simulink model "os_polyphase_synth_4096c"  (R2021a) (continued)
== system os_polyphase_synth_4096c/pfb_fir/pol1_in1_tap77 == (continued)
      | -PCIN>>17+P+(D-A)*(D-A)
      | -PCIN>>17+P+(D-A)*B
      | -PCIN>>17+P+(D-A)*BCIN
      | -PCIN>>17+P+(D-ACIN)
      | -PCIN>>17+P+(D-ACIN)*(D-ACIN)
      | -PCIN>>17+P+(D-ACIN)*B
      | -PCIN>>17+P+(D-ACIN)*BCIN
      | -PCIN>>17+P+A
      | -PCIN>>17+P+A*A
      | -PCIN>>17+P+A*B
      | -PCIN>>17+P+A*BCIN
      | -PCIN>>17+P+ACIN
      | -PCIN>>17+P+ACIN*ACIN
      | -PCIN>>17+P+ACIN*B
      | -PCIN>>17+P+ACIN*BCIN
      | -PCIN>>17+P+B*(A+D)
      | -PCIN>>17+P+B*(ACIN+D)
      | -PCIN>>17+P+B*(D+A)
      | -PCIN>>17+P+B*(D+ACIN)
      | -PCIN>>17+P+B*(D-A)
      | -PCIN>>17+P+B*(D-ACIN)
      | -PCIN>>17+P+B*A
      | -PCIN>>17+P+B*ACIN
      | -PCIN>>17+P+B*D
      | -PCIN>>17+P+BCIN*(A+D)
      | -PCIN>>17+P+BCIN*(ACIN+D)
      | -PCIN>>17+P+BCIN*(D+A)
      | -PCIN>>17+P+BCIN*(D+ACIN)
      | -PCIN>>17+P+BCIN*(D-A)
      | -PCIN>>17+P+BCIN*(D-ACIN)
      | -PCIN>>17+P+BCIN*A
      | -PCIN>>17+P+BCIN*ACIN
      | -PCIN>>17+P+BCIN*D
      | -PCIN>>17+P+C
      | -PCIN>>17+P+C+C
      | -PCIN>>17+P+C+CONCAT
      | -PCIN>>17+P+C+P
      | -PCIN>>17+P+CONCAT
      | -PCIN>>17+P+CONCAT+C
      | -PCIN>>17+P+D
      | -PCIN>>17+P+D*B
      | -PCIN>>17+P+D*BCIN
      | -PCIN>>17+P+D*D
      | -PCIN>>17+P+P
      | -PCIN>>17+P+P+C
      | -PCIN>>17-((A+D))
      | -PCIN>>17-((A+D)*(A+D))
      | -PCIN>>17-((A+D)*B)
      | -PCIN>>17-((A+D)*BCIN)
      | -PCIN>>17-((ACIN+D))
      | -PCIN>>17-((ACIN+D)*(ACIN+D))
      | -PCIN>>17-((ACIN+D)*B)
      | -PCIN>>17-((ACIN+D)*BCIN)
      | -PCIN>>17-((D+A))
      | -PCIN>>17-((D+A)*(D+A))
      | -PCIN>>17-((D+A)*B)
      | -PCIN>>17-((D+A)*BCIN)
      | -PCIN>>17-((D+ACIN))
      | -PCIN>>17-((D+ACIN)*(D+ACIN))
      | -PCIN>>17-((D+ACIN)*B)
      | -PCIN>>17-((D+ACIN)*BCIN)
      | -PCIN>>17-((D-A))
      | -PCIN>>17-((D-A)*(D-A))
      | -PCIN>>17-((D-A)*B)
      | -PCIN>>17-((D-A)*BCIN)
      | -PCIN>>17-((D-ACIN))
      | -PCIN>>17-((D-ACIN)*(D-ACIN))
      | -PCIN>>17-((D-ACIN)*B)
      | -PCIN>>17-((D-ACIN)*BCIN)
      | -PCIN>>17-(A)
      | -PCIN>>17-(A*A)
      | -PCIN>>17-(A*B)
      | -PCIN>>17-(A*BCIN)
      | -PCIN>>17-(ACIN)
      | -PCIN>>17-(ACIN*ACIN)
      | -PCIN>>17-(ACIN*B)
      | -PCIN>>17-(ACIN*BCIN)
      | -PCIN>>17-(B*(A+D))
      | -PCIN>>17-(B*(ACIN+D))
      | -PCIN>>17-(B*(D+A))
      | -PCIN>>17-(B*(D+ACIN))
      | -PCIN>>17-(B*(D-A))
      | -PCIN>>17-(B*(D-ACIN))
      | -PCIN>>17-(B*A)
      | -PCIN>>17-(B*ACIN)
      | -PCIN>>17-(B*D)
      | -PCIN>>17-(BCIN*(A+D))
      | -PCIN>>17-(BCIN*(ACIN+D))
      | -PCIN>>17-(BCIN*(D+A))
      | -PCIN>>17-(BCIN*(D+ACIN))
      | -PCIN>>17-(BCIN*(D-A))
      | -PCIN>>17-(BCIN*(D-ACIN))
      | -PCIN>>17-(BCIN*A)
      | -PCIN>>17-(BCIN*ACIN)
      | -PCIN>>17-(BCIN*D)
      | -PCIN>>17-(D)
      | -PCIN>>17-(D*B)
      | -PCIN>>17-(D*BCIN)
      | -PCIN>>17-(D*D)
      | 0
      | A
      | A*A
      | A*A+C
      | A*A+C+C
      | A*A+C+C+CARRYIN
      | A*A+C+CARRYIN
      | A*A+C+P
      | A*A+C+P+CARRYIN
      | A*A+C+P>>17
      | A*A+C+P>>17+CARRYIN
      | A*A+C+PCIN
      | A*A+C+PCIN+CARRYIN
      | A*A+C+PCIN>>17
      | A*A+C+PCIN>>17+CARRYIN
      | A*A+C-C
      | A*A+C-P
      | A*A+C-P>>17
      | A*A+C-PCIN
      | A*A+C-PCIN>>17
      | A*A+CARRYIN
      | A*A+P
      | A*A+P+C
      | A*A+P+C+CARRYIN
      | A*A+P+CARRYIN
      | A*A+P+P
      | A*A+P+P+CARRYIN
      | A*A+P+P>>17
      | A*A+P+P>>17+CARRYIN
      | A*A+P+PCIN
      | A*A+P+PCIN+CARRYIN
      | A*A+P+PCIN>>17
      | A*A+P+PCIN>>17+CARRYIN
      | A*A+P-C
      | A*A+P-P
      | A*A+P-P>>17
      | A*A+P-PCIN
      | A*A+P-PCIN>>17
      | A*A+P>>17
      | A*A+P>>17+C
      | A*A+P>>17+C+CARRYIN
      | A*A+P>>17+CARRYIN
      | A*A+P>>17+P
      | A*A+P>>17+P+CARRYIN
      | A*A+PCIN
      | A*A+PCIN+C
      | A*A+PCIN+C+CARRYIN
      | A*A+PCIN+CARRYIN
      | A*A+PCIN+P
      | A*A+PCIN+P+CARRYIN
      | A*A+PCIN>>17
      | A*A+PCIN>>17+C
      | A*A+PCIN>>17+C+CARRYIN
      | A*A+PCIN>>17+CARRYIN
      | A*A+PCIN>>17+P
      | A*A+PCIN>>17+P+CARRYIN
      | A*A-C
      | A*A-P
      | A*A-P>>17
      | A*A-PCIN
      | A*A-PCIN>>17
      | A*B
      | A*B+C
      | A*B+C+C
      | A*B+C+C+CARRYIN
      | A*B+C+CARRYIN
      | A*B+C+P
      | A*B+C+P+CARRYIN
      | A*B+C+P>>17
      | A*B+C+P>>17+CARRYIN
      | A*B+C+PCIN
      | A*B+C+PCIN+CARRYIN
      | A*B+C+PCIN>>17
      | A*B+C+PCIN>>17+CARRYIN
      | A*B+C-C
      | A*B+C-P
      | A*B+C-P>>17
      | A*B+C-PCIN
      | A*B+C-PCIN>>17
      | A*B+CARRYIN
      | A*B+P
      | A*B+P+C
      | A*B+P+C+CARRYIN
      | A*B+P+CARRYIN
      | A*B+P+P
      | A*B+P+P+CARRYIN
      | A*B+P+P>>17
      | A*B+P+P>>17+CARRYIN
      | A*B+P+PCIN
      | A*B+P+PCIN+CARRYIN
      | A*B+P+PCIN>>17
      | A*B+P+PCIN>>17+CARRYIN
      | A*B+P-C
      | A*B+P-P
      | A*B+P-P>>17
      | A*B+P-PCIN
      | A*B+P-PCIN>>17
      | A*B+P>>17
      | A*B+P>>17+C
      | A*B+P>>17+C+CARRYIN
      | A*B+P>>17+CARRYIN
      | A*B+P>>17+P
      | A*B+P>>17+P+CARRYIN
      | A*B+PCIN
      | A*B+PCIN+C
      | A*B+PCIN+C+CARRYIN
      | A*B+PCIN+CARRYIN
      | A*B+PCIN+P
      | A*B+PCIN+P+CARRYIN
      | A*B+PCIN>>17
      | A*B+PCIN>>17+C
      | A*B+PCIN>>17+C+CARRYIN
      | A*B+PCIN>>17+CARRYIN
      | A*B+PCIN>>17+P
      | A*B+PCIN>>17+P+CARRYIN
      | A*B-C
      | A*B-P
      | A*B-P>>17
      | A*B-PCIN
      | A*B-PCIN>>17
      | A*BCIN
      | A*BCIN+C
      | A*BCIN+C+C
      | A*BCIN+C+C+CARRYIN
      | A*BCIN+C+CARRYIN
      | A*BCIN+C+P
      | A*BCIN+C+P+CARRYIN
      | A*BCIN+C+P>>17
      | A*BCIN+C+P>>17+CARRYIN
      | A*BCIN+C+PCIN
      | A*BCIN+C+PCIN+CARRYIN
      | A*BCIN+C+PCIN>>17
      | A*BCIN+C+PCIN>>17+CARRYIN
      | A*BCIN+C-C
      | A*BCIN+C-P
      | A*BCIN+C-P>>17
      | A*BCIN+C-PCIN
      | A*BCIN+C-PCIN>>17
      | A*BCIN+CARRYIN
      | A*BCIN+P
      | A*BCIN+P+C
      | A*BCIN+P+C+CARRYIN
      | A*BCIN+P+CARRYIN
      | A*BCIN+P+P
      | A*BCIN+P+P+CARRYIN
      | A*BCIN+P+P>>17
      | A*BCIN+P+P>>17+CARRYIN
      | A*BCIN+P+PCIN
      | A*BCIN+P+PCIN+CARRYIN
      | A*BCIN+P+PCIN>>17
      | A*BCIN+P+PCIN>>17+CARRYIN
      | A*BCIN+P-C
      | A*BCIN+P-P
      | A*BCIN+P-P>>17
      | A*BCIN+P-PCIN
      | A*BCIN+P-PCIN>>17
      | A*BCIN+P>>17
      | A*BCIN+P>>17+C
      | A*BCIN+P>>17+C+CARRYIN
      | A*BCIN+P>>17+CARRYIN
      | A*BCIN+P>>17+P
      | A*BCIN+P>>17+P+CARRYIN
      | A*BCIN+PCIN
      | A*BCIN+PCIN+C
      | A*BCIN+PCIN+C+CARRYIN
      | A*BCIN+PCIN+CARRYIN
      | A*BCIN+PCIN+P
      | A*BCIN+PCIN+P+CARRYIN
      | A*BCIN+PCIN>>17
      | A*BCIN+PCIN>>17+C
      | A*BCIN+PCIN>>17+C+CARRYIN
      | A*BCIN+PCIN>>17+CARRYIN
      | A*BCIN+PCIN>>17+P
      | A*BCIN+PCIN>>17+P+CARRYIN
      | A*BCIN-C
      | A*BCIN-P
      | A*BCIN-P>>17
      | A*BCIN-PCIN
      | A*BCIN-PCIN>>17
      | A+C
      | A+C+C
      | A+C+C+CARRYIN
      | A+C+CARRYIN
      | A+C+P
      | A+C+P+CARRYIN
      | A+C+P>>17
      | A+C+P>>17+CARRYIN
      | A+C+PCIN
      | A+C+PCIN+CARRYIN
      | A+C+PCIN>>17
      | A+C+PCIN>>17+CARRYIN
      | A+C-C
      | A+C-P
      | A+C-P>>17
      | A+C-PCIN
      | A+C-PCIN>>17
      | A+CARRYIN
      | A+P
      | A+P+C
      | A+P+C+CARRYIN
      | A+P+CARRYIN
      | A+P+P
      | A+P+P+CARRYIN
      | A+P+P>>17
      | A+P+P>>17+CARRYIN
      | A+P+PCIN
      | A+P+PCIN+CARRYIN
      | A+P+PCIN>>17
      | A+P+PCIN>>17+CARRYIN
      | A+P-C
      | A+P-P
      | A+P-P>>17
      | A+P-PCIN
      | A+P-PCIN>>17
      | A+P>>17
      | A+P>>17+C
      | A+P>>17+C+CARRYIN
      | A+P>>17+CARRYIN
      | A+P>>17+P
      | A+P>>17+P+CARRYIN
      | A+PCIN
      | A+PCIN+C
      | A+PCIN+C+CARRYIN
      | A+PCIN+CARRYIN
      | A+PCIN+P
      | A+PCIN+P+CARRYIN
      | A+PCIN>>17
      | A+PCIN>>17+C
      | A+PCIN>>17+C+CARRYIN
      | A+PCIN>>17+CARRYIN
      | A+PCIN>>17+P
      | A+PCIN>>17+P+CARRYIN
      | A-C
      | A-P
      | A-P>>17
      | A-PCIN
      | A-PCIN>>17
      | ACIN
      | ACIN*ACIN
      | ACIN*ACIN+C
      | ACIN*ACIN+C+C
      | ACIN*ACIN+C+C+CARRYIN
      | ACIN*ACIN+C+CARRYIN
      | ACIN*ACIN+C+P
      | ACIN*ACIN+C+P+CARRYIN
      | ACIN*ACIN+C+P>>17
      | ACIN*ACIN+C+P>>17+CARRYIN
      | ACIN*ACIN+C+PCIN
      | ACIN*ACIN+C+PCIN+CARRYIN
      | ACIN*ACIN+C+PCIN>>17
      | ACIN*ACIN+C+PCIN>>17+CARRYIN
      | ACIN*ACIN+C-C
      | ACIN*ACIN+C-P
      | ACIN*ACIN+C-P>>17
      | ACIN*ACIN+C-PCIN
      | ACIN*ACIN+C-PCIN>>17
      | ACIN*ACIN+CARRYIN
      | ACIN*ACIN+P
      | ACIN*ACIN+P+C
      | ACIN*ACIN+P+C+CARRYIN
      | ACIN*ACIN+P+CARRYIN
      | ACIN*ACIN+P+P
      | ACIN*ACIN+P+P+CARRYIN
      | ACIN*ACIN+P+P>>17
      | ACIN*ACIN+P+P>>17+CARRYIN
      | ACIN*ACIN+P+PCIN
      | ACIN*ACIN+P+PCIN+CARRYIN
      | ACIN*ACIN+P+PCIN>>17
      | ACIN*ACIN+P+PCIN>>17+CARRYIN
      | ACIN*ACIN+P-C
      | ACIN*ACIN+P-P
      | ACIN*ACIN+P-P>>17
      | ACIN*ACIN+P-PCIN
      | ACIN*ACIN+P-PCIN>>17
      | ACIN*ACIN+P>>17
      | ACIN*ACIN+P>>17+C
      | ACIN*ACIN+P>>17+C+CARRYIN
      | ACIN*ACIN+P>>17+CARRYIN
      | ACIN*ACIN+P>>17+P
      | ACIN*ACIN+P>>17+P+CARRYIN
      | ACIN*ACIN+PCIN
      | ACIN*ACIN+PCIN+C
      | ACIN*ACIN+PCIN+C+CARRYIN
      | ACIN*ACIN+PCIN+CARRYIN
      | ACIN*ACIN+PCIN+P
      | ACIN*ACIN+PCIN+P+CARRYIN
      | ACIN*ACIN+PCIN>>17
      | ACIN*ACIN+PCIN>>17+C
      | ACIN*ACIN+PCIN>>17+C+CARRYIN
      | ACIN*ACIN+PCIN>>17+CARRYIN
      | ACIN*ACIN+PCIN>>17+P
      | ACIN*ACIN+PCIN>>17+P+CARRYIN
      | ACIN*ACIN-C
      | ACIN*ACIN-P
      | ACIN*ACIN-P>>17
      | ACIN*ACIN-PCIN
      | ACIN*ACIN-PCIN>>17
      | ACIN*B
      | ACIN*B+C
      | ACIN*B+C+C
      | ACIN*B+C+C+CARRYIN
      | ACIN*B+C+CARRYIN
      | ACIN*B+C+P
      | ACIN*B+C+P+CARRYIN
      | ACIN*B+C+P>>17
      | ACIN*B+C+P>>17+CARRYIN
      | ACIN*B+C+PCIN
      | ACIN*B+C+PCIN+CARRYIN
      | ACIN*B+C+PCIN>>17
      | ACIN*B+C+PCIN>>17+CARRYIN
      | ACIN*B+C-C
      | ACIN*B+C-P
      | ACIN*B+C-P>>17
      | ACIN*B+C-PCIN
      | ACIN*B+C-PCIN>>17
      | ACIN*B+CARRYIN
      | ACIN*B+P
      | ACIN*B+P+C
      | ACIN*B+P+C+CARRYIN
      | ACIN*B+P+CARRYIN
      | ACIN*B+P+P
      | ACIN*B+P+P+CARRYIN
      | ACIN*B+P+P>>17
      | ACIN*B+P+P>>17+CARRYIN
      | ACIN*B+P+PCIN
      | ACIN*B+P+PCIN+CARRYIN
      | ACIN*B+P+PCIN>>17
      | ACIN*B+P+PCIN>>17+CARRYIN
      | ACIN*B+P-C
      | ACIN*B+P-P
      | ACIN*B+P-P>>17
      | ACIN*B+P-PCIN
      | ACIN*B+P-PCIN>>17
      | ACIN*B+P>>17
      | ACIN*B+P>>17+C
      | ACIN*B+P>>17+C+CARRYIN
      | ACIN*B+P>>17+CARRYIN
      | ACIN*B+P>>17+P
      | ACIN*B+P>>17+P+CARRYIN
      | ACIN*B+PCIN
      | ACIN*B+PCIN+C
      | ACIN*B+PCIN+C+CARRYIN
      | ACIN*B+PCIN+CARRYIN
      | ACIN*B+PCIN+P
      | ACIN*B+PCIN+P+CARRYIN
      | ACIN*B+PCIN>>17
      | ACIN*B+PCIN>>17+C
      | ACIN*B+PCIN>>17+C+CARRYIN
      | ACIN*B+PCIN>>17+CARRYIN
      | ACIN*B+PCIN>>17+P
      | ACIN*B+PCIN>>17+P+CARRYIN
      | ACIN*B-C
      | ACIN*B-P
      | ACIN*B-P>>17
      | ACIN*B-PCIN
      | ACIN*B-PCIN>>17
      | ACIN*BCIN
      | ACIN*BCIN+C
      | ACIN*BCIN+C+C
      | ACIN*BCIN+C+C+CARRYIN
      | ACIN*BCIN+C+CARRYIN
      | ACIN*BCIN+C+P
      | ACIN*BCIN+C+P+CARRYIN
      | ACIN*BCIN+C+P>>17
      | ACIN*BCIN+C+P>>17+CARRYIN
      | ACIN*BCIN+C+PCIN
      | ACIN*BCIN+C+PCIN+CARRYIN
      | ACIN*BCIN+C+PCIN>>17
      | ACIN*BCIN+C+PCIN>>17+CARRYIN
      | ACIN*BCIN+C-C
      | ACIN*BCIN+C-P
      | ACIN*BCIN+C-P>>17
      | ACIN*BCIN+C-PCIN
      | ACIN*BCIN+C-PCIN>>17
      | ACIN*BCIN+CARRYIN
      | ACIN*BCIN+P
      | ACIN*BCIN+P+C
      | ACIN*BCIN+P+C+CARRYIN
      | ACIN*BCIN+P+CARRYIN
      | ACIN*BCIN+P+P
      | ACIN*BCIN+P+P+CARRYIN
      | ACIN*BCIN+P+P>>17
      | ACIN*BCIN+P+P>>17+CARRYIN
      | ACIN*BCIN+P+PCIN
      | ACIN*BCIN+P+PCIN+CARRYIN
      | ACIN*BCIN+P+PCIN>>17
      | ACIN*BCIN+P+PCIN>>17+CARRYIN
      | ACIN*BCIN+P-C
      | ACIN*BCIN+P-P
      | ACIN*BCIN+P-P>>17
      | ACIN*BCIN+P-PCIN
      | ACIN*BCIN+P-PCIN>>17
      | ACIN*BCIN+P>>17
      | ACIN*BCIN+P>>17+C
      | ACIN*BCIN+P>>17+C+CARRYIN
      | ACIN*BCIN+P>>17+CARRYIN
      | ACIN*BCIN+P>>17+P
      | ACIN*BCIN+P>>17+P+CARRYIN
      | ACIN*BCIN+PCIN
      | ACIN*BCIN+PCIN+C
      | ACIN*BCIN+PCIN+C+CARRYIN
      | ACIN*BCIN+PCIN+CARRYIN
      | ACIN*BCIN+PCIN+P
      | ACIN*BCIN+PCIN+P+CARRYIN
      | ACIN*BCIN+PCIN>>17
      | ACIN*BCIN+PCIN>>17+C
      | ACIN*BCIN+PCIN>>17+C+CARRYIN
      | ACIN*BCIN+PCIN>>17+CARRYIN
      | ACIN*BCIN+PCIN>>17+P
      | ACIN*BCIN+PCIN>>17+P+CARRYIN
      | ACIN*BCIN-C
      | ACIN*BCIN-P
      | ACIN*BCIN-P>>17
      | ACIN*BCIN-PCIN
      | ACIN*BCIN-PCIN>>17
      | ACIN+C
      | ACIN+C+C
      | ACIN+C+C+CARRYIN
      | ACIN+C+CARRYIN
      | ACIN+C+P
      | ACIN+C+P+CARRYIN
      | ACIN+C+P>>17
      | ACIN+C+P>>17+CARRYIN
      | ACIN+C+PCIN
      | ACIN+C+PCIN+CARRYIN
      | ACIN+C+PCIN>>17
      | ACIN+C+PCIN>>17+CARRYIN
      | ACIN+C-C
      | ACIN+C-P
      | ACIN+C-P>>17
      | ACIN+C-PCIN
      | ACIN+C-PCIN>>17
      | ACIN+CARRYIN
      | ACIN+P
      | ACIN+P+C
      | ACIN+P+C+CARRYIN
      | ACIN+P+CARRYIN
      | ACIN+P+P
      | ACIN+P+P+CARRYIN
      | ACIN+P+P>>17
      | ACIN+P+P>>17+CARRYIN
      | ACIN+P+PCIN
      | ACIN+P+PCIN+CARRYIN
      | ACIN+P+PCIN>>17
      | ACIN+P+PCIN>>17+CARRYIN
      | ACIN+P-C
      | ACIN+P-P
      | ACIN+P-P>>17
      | ACIN+P-PCIN
      | ACIN+P-PCIN>>17
      | ACIN+P>>17
      | ACIN+P>>17+C
      | ACIN+P>>17+C+CARRYIN
      | ACIN+P>>17+CARRYIN
      | ACIN+P>>17+P
      | ACIN+P>>17+P+CARRYIN
      | ACIN+PCIN
      | ACIN+PCIN+C
      | ACIN+PCIN+C+CARRYIN
      | ACIN+PCIN+CARRYIN
      | ACIN+PCIN+P
      | ACIN+PCIN+P+CARRYIN
      | ACIN+PCIN>>17
      | ACIN+PCIN>>17+C
      | ACIN+PCIN>>17+C+CARRYIN
      | ACIN+PCIN>>17+CARRYIN
      | ACIN+PCIN>>17+P
      | ACIN+PCIN>>17+P+CARRYIN
      | ACIN-C
      | ACIN-P
      | ACIN-P>>17
      | ACIN-PCIN
      | ACIN-PCIN>>17
      | B*(A+D)
      | B*(A+D)+C
      | B*(A+D)+C+C
      | B*(A+D)+C+C+CARRYIN
      | B*(A+D)+C+CARRYIN
      | B*(A+D)+C+P
      | B*(A+D)+C+P+CARRYIN
      | B*(A+D)+C+P>>17
      | B*(A+D)+C+P>>17+CARRYIN
      | B*(A+D)+C+PCIN
      | B*(A+D)+C+PCIN+CARRYIN
      | B*(A+D)+C+PCIN>>17
      | B*(A+D)+C+PCIN>>17+CARRYIN
      | B*(A+D)+C-C
      | B*(A+D)+C-P
      | B*(A+D)+C-P>>17
      | B*(A+D)+C-PCIN
      | B*(A+D)+C-PCIN>>17
      | B*(A+D)+CARRYIN
      | B*(A+D)+P
      | B*(A+D)+P+C
      | B*(A+D)+P+C+CARRYIN
      | B*(A+D)+P+CARRYIN
      | B*(A+D)+P+P
      | B*(A+D)+P+P+CARRYIN
      | B*(A+D)+P+P>>17
      | B*(A+D)+P+P>>17+CARRYIN
      | B*(A+D)+P+PCIN
      | B*(A+D)+P+PCIN+CARRYIN
      | B*(A+D)+P+PCIN>>17
      | B*(A+D)+P+PCIN>>17+CARRYIN
      | B*(A+D)+P-C
      | B*(A+D)+P-P
      | B*(A+D)+P-P>>17
      | B*(A+D)+P-PCIN
      | B*(A+D)+P-PCIN>>17
      | B*(A+D)+P>>17
      | B*(A+D)+P>>17+C
      | B*(A+D)+P>>17+C+CARRYIN
      | B*(A+D)+P>>17+CARRYIN
      | B*(A+D)+P>>17+P
      | B*(A+D)+P>>17+P+CARRYIN
      | B*(A+D)+PCIN
      | B*(A+D)+PCIN+C
      | B*(A+D)+PCIN+C+CARRYIN
      | B*(A+D)+PCIN+CARRYIN
      | B*(A+D)+PCIN+P
      | B*(A+D)+PCIN+P+CARRYIN
      | B*(A+D)+PCIN>>17
      | B*(A+D)+PCIN>>17+C
      | B*(A+D)+PCIN>>17+C+CARRYIN
      | B*(A+D)+PCIN>>17+CARRYIN
      | B*(A+D)+PCIN>>17+P
      | B*(A+D)+PCIN>>17+P+CARRYIN
      | B*(A+D)-C
      | B*(A+D)-P
      | B*(A+D)-P>>17
      | B*(A+D)-PCIN
      | B*(A+D)-PCIN>>17
      | B*(ACIN+D)
      | B*(ACIN+D)+C
      | B*(ACIN+D)+C+C
      | B*(ACIN+D)+C+C+CARRYIN
      | B*(ACIN+D)+C+CARRYIN
      | B*(ACIN+D)+C+P
      | B*(ACIN+D)+C+P+CARRYIN
      | B*(ACIN+D)+C+P>>17
      | B*(ACIN+D)+C+P>>17+CARRYIN
      | B*(ACIN+D)+C+PCIN
      | B*(ACIN+D)+C+PCIN+CARRYIN
      | B*(ACIN+D)+C+PCIN>>17
      | B*(ACIN+D)+C+PCIN>>17+CARRYIN
      | B*(ACIN+D)+C-C
      | B*(ACIN+D)+C-P
      | B*(ACIN+D)+C-P>>17
      | B*(ACIN+D)+C-PCIN
      | B*(ACIN+D)+C-PCIN>>17
      | B*(ACIN+D)+CARRYIN
      | B*(ACIN+D)+P
      | B*(ACIN+D)+P+C
      | B*(ACIN+D)+P+C+CARRYIN
      | B*(ACIN+D)+P+CARRYIN
      | B*(ACIN+D)+P+P
      | B*(ACIN+D)+P+P+CARRYIN
      | B*(ACIN+D)+P+P>>17
      | B*(ACIN+D)+P+P>>17+CARRYIN
      | B*(ACIN+D)+P+PCIN
      | B*(ACIN+D)+P+PCIN+CARRYIN
      | B*(ACIN+D)+P+PCIN>>17
      | B*(ACIN+D)+P+PCIN>>17+CARRYIN
      | B*(ACIN+D)+P-C
      | B*(ACIN+D)+P-P
      | B*(ACIN+D)+P-P>>17
      | B*(ACIN+D)+P-PCIN
      | B*(ACIN+D)+P-PCIN>>17
      | B*(ACIN+D)+P>>17
      | B*(ACIN+D)+P>>17+C
      | B*(ACIN+D)+P>>17+C+CARRYIN
      | B*(ACIN+D)+P>>17+CARRYIN
      | B*(ACIN+D)+P>>17+P
      | B*(ACIN+D)+P>>17+P+CARRYIN
      | B*(ACIN+D)+PCIN
      | B*(ACIN+D)+PCIN+C
      | B*(ACIN+D)+PCIN+C+CARRYIN
      | B*(ACIN+D)+PCIN+CARRYIN
      | B*(ACIN+D)+PCIN+P
      | B*(ACIN+D)+PCIN+P+CARRYIN
      | B*(ACIN+D)+PCIN>>17
      | B*(ACIN+D)+PCIN>>17+C
      | B*(ACIN+D)+PCIN>>17+C+CARRYIN
      | B*(ACIN+D)+PCIN>>17+CARRYIN
      | B*(ACIN+D)+PCIN>>17+P
      | B*(ACIN+D)+PCIN>>17+P+CARRYIN
      | B*(ACIN+D)-C
      | B*(ACIN+D)-P
      | B*(ACIN+D)-P>>17
      | B*(ACIN+D)-PCIN
      | B*(ACIN+D)-PCIN>>17
      | B*(D+A)
      | B*(D+A)+C
      | B*(D+A)+C+C
      | B*(D+A)+C+C+CARRYIN
      | B*(D+A)+C+CARRYIN
      | B*(D+A)+C+P
      | B*(D+A)+C+P+CARRYIN
      | B*(D+A)+C+P>>17
      | B*(D+A)+C+P>>17+CARRYIN
      | B*(D+A)+C+PCIN
      | B*(D+A)+C+PCIN+CARRYIN
      | B*(D+A)+C+PCIN>>17
      | B*(D+A)+C+PCIN>>17+CARRYIN
      | B*(D+A)+C-C
      | B*(D+A)+C-P
      | B*(D+A)+C-P>>17
      | B*(D+A)+C-PCIN
      | B*(D+A)+C-PCIN>>17
      | B*(D+A)+CARRYIN
      | B*(D+A)+P
      | B*(D+A)+P+C
      | B*(D+A)+P+C+CARRYIN
      | B*(D+A)+P+CARRYIN
      | B*(D+A)+P+P
      | B*(D+A)+P+P+CARRYIN
      | B*(D+A)+P+P>>17
      | B*(D+A)+P+P>>17+CARRYIN
      | B*(D+A)+P+PCIN
      | B*(D+A)+P+PCIN+CARRYIN
      | B*(D+A)+P+PCIN>>17
      | B*(D+A)+P+PCIN>>17+CARRYIN
      | B*(D+A)+P-C
      | B*(D+A)+P-P
      | B*(D+A)+P-P>>17
      | B*(D+A)+P-PCIN
      | B*(D+A)+P-PCIN>>17
      | B*(D+A)+P>>17
      | B*(D+A)+P>>17+C
      | B*(D+A)+P>>17+C+CARRYIN
      | B*(D+A)+P>>17+CARRYIN
      | B*(D+A)+P>>17+P
      | B*(D+A)+P>>17+P+CARRYIN
      | B*(D+A)+PCIN
      | B*(D+A)+PCIN+C
      | B*(D+A)+PCIN+C+CARRYIN
      | B*(D+A)+PCIN+CARRYIN
      | B*(D+A)+PCIN+P
      | B*(D+A)+PCIN+P+CARRYIN
      | B*(D+A)+PCIN>>17
      | B*(D+A)+PCIN>>17+C
      | B*(D+A)+PCIN>>17+C+CARRYIN
      | B*(D+A)+PCIN>>17+CARRYIN
      | B*(D+A)+PCIN>>17+P
      | B*(D+A)+PCIN>>17+P+CARRYIN
      | B*(D+A)-C
      | B*(D+A)-P
      | B*(D+A)-P>>17
      | B*(D+A)-PCIN
      | B*(D+A)-PCIN>>17
      | B*(D+ACIN)
      | B*(D+ACIN)+C
      | B*(D+ACIN)+C+C
      | B*(D+ACIN)+C+C+CARRYIN
      | B*(D+ACIN)+C+CARRYIN
      | B*(D+ACIN)+C+P
      | B*(D+ACIN)+C+P+CARRYIN
      | B*(D+ACIN)+C+P>>17
      | B*(D+ACIN)+C+P>>17+CARRYIN
      | B*(D+ACIN)+C+PCIN
      | B*(D+ACIN)+C+PCIN+CARRYIN
      | B*(D+ACIN)+C+PCIN>>17
      | B*(D+ACIN)+C+PCIN>>17+CARRYIN
      | B*(D+ACIN)+C-C
      | B*(D+ACIN)+C-P
      | B*(D+ACIN)+C-P>>17
      | B*(D+ACIN)+C-PCIN
      | B*(D+ACIN)+C-PCIN>>17
      | B*(D+ACIN)+CARRYIN
      | B*(D+ACIN)+P
      | B*(D+ACIN)+P+C
      | B*(D+ACIN)+P+C+CARRYIN
      | B*(D+ACIN)+P+CARRYIN
      | B*(D+ACIN)+P+P
      | B*(D+ACIN)+P+P+CARRYIN
      | B*(D+ACIN)+P+P>>17
      | B*(D+ACIN)+P+P>>17+CARRYIN
      | B*(D+ACIN)+P+PCIN
      | B*(D+ACIN)+P+PCIN+CARRYIN
      | B*(D+ACIN)+P+PCIN>>17
      | B*(D+ACIN)+P+PCIN>>17+CARRYIN
      | B*(D+ACIN)+P-C
      | B*(D+ACIN)+P-P
      | B*(D+ACIN)+P-P>>17
      | B*(D+ACIN)+P-PCIN
      | B*(D+ACIN)+P-PCIN>>17
      | B*(D+ACIN)+P>>17
      | B*(D+ACIN)+P>>17+C
      | B*(D+ACIN)+P>>17+C+CARRYIN
      | B*(D+ACIN)+P>>17+CARRYIN
      | B*(D+ACIN)+P>>17+P
      | B*(D+ACIN)+P>>17+P+CARRYIN
      | B*(D+ACIN)+PCIN
      | B*(D+ACIN)+PCIN+C
      | B*(D+ACIN)+PCIN+C+CARRYIN
      | B*(D+ACIN)+PCIN+CARRYIN
      | B*(D+ACIN)+PCIN+P
      | B*(D+ACIN)+PCIN+P+CARRYIN
      | B*(D+ACIN)+PCIN>>17
      | B*(D+ACIN)+PCIN>>17+C
      | B*(D+ACIN)+PCIN>>17+C+CARRYIN
      | B*(D+ACIN)+PCIN>>17+CARRYIN
      | B*(D+ACIN)+PCIN>>17+P
      | B*(D+ACIN)+PCIN>>17+P+CARRYIN
      | B*(D+ACIN)-C
      | B*(D+ACIN)-P
      | B*(D+ACIN)-P>>17
      | B*(D+ACIN)-PCIN
      | B*(D+ACIN)-PCIN>>17
      | B*(D-A)
      | B*(D-A)+C
      | B*(D-A)+C+C
      | B*(D-A)+C+C+CARRYIN
      | B*(D-A)+C+CARRYIN
      | B*(D-A)+C+P
      | B*(D-A)+C+P+CARRYIN
      | B*(D-A)+C+P>>17
      | B*(D-A)+C+P>>17+CARRYIN
      | B*(D-A)+C+PCIN
      | B*(D-A)+C+PCIN+CARRYIN
      | B*(D-A)+C+PCIN>>17
      | B*(D-A)+C+PCIN>>17+CARRYIN
      | B*(D-A)+C-C
      | B*(D-A)+C-P
      | B*(D-A)+C-P>>17
      | B*(D-A)+C-PCIN
      | B*(D-A)+C-PCIN>>17
      | B*(D-A)+CARRYIN
      | B*(D-A)+P
      | B*(D-A)+P+C
      | B*(D-A)+P+C+CARRYIN
      | B*(D-A)+P+CARRYIN
      | B*(D-A)+P+P
      | B*(D-A)+P+P+CARRYIN
      | B*(D-A)+P+P>>17
      | B*(D-A)+P+P>>17+CARRYIN
      | B*(D-A)+P+PCIN
      | B*(D-A)+P+PCIN+CARRYIN
      | B*(D-A)+P+PCIN>>17
      | B*(D-A)+P+PCIN>>17+CARRYIN
      | B*(D-A)+P-C
      | B*(D-A)+P-P
      | B*(D-A)+P-P>>17
      | B*(D-A)+P-PCIN
      | B*(D-A)+P-PCIN>>17
      | B*(D-A)+P>>17
      | B*(D-A)+P>>17+C
      | B*(D-A)+P>>17+C+CARRYIN
      | B*(D-A)+P>>17+CARRYIN
      | B*(D-A)+P>>17+P
      | B*(D-A)+P>>17+P+CARRYIN
      | B*(D-A)+PCIN
      | B*(D-A)+PCIN+C
      | B*(D-A)+PCIN+C+CARRYIN
      | B*(D-A)+PCIN+CARRYIN
      | B*(D-A)+PCIN+P
      | B*(D-A)+PCIN+P+CARRYIN
      | B*(D-A)+PCIN>>17
      | B*(D-A)+PCIN>>17+C
      | B*(D-A)+PCIN>>17+C+CARRYIN
      | B*(D-A)+PCIN>>17+CARRYIN
      | B*(D-A)+PCIN>>17+P
      | B*(D-A)+PCIN>>17+P+CARRYIN
      | B*(D-A)-C
      | B*(D-A)-P
      | B*(D-A)-P>>17
      | B*(D-A)-PCIN
      | B*(D-A)-PCIN>>17
      | B*(D-ACIN)
      | B*(D-ACIN)+C
      | B*(D-ACIN)+C+C
      | B*(D-ACIN)+C+C+CARRYIN
      | B*(D-ACIN)+C+CARRYIN
      | B*(D-ACIN)+C+P
      | B*(D-ACIN)+C+P+CARRYIN
      | B*(D-ACIN)+C+P>>17
      | B*(D-ACIN)+C+P>>17+CARRYIN
      | B*(D-ACIN)+C+PCIN
      | B*(D-ACIN)+C+PCIN+CARRYIN
      | B*(D-ACIN)+C+PCIN>>17
      | B*(D-ACIN)+C+PCIN>>17+CARRYIN
      | B*(D-ACIN)+C-C
      | B*(D-ACIN)+C-P
      | B*(D-ACIN)+C-P>>17
      | B*(D-ACIN)+C-PCIN
      | B*(D-ACIN)+C-PCIN>>17
      | B*(D-ACIN)+CARRYIN
      | B*(D-ACIN)+P
      | B*(D-ACIN)+P+C
      | B*(D-ACIN)+P+C+CARRYIN
      | B*(D-ACIN)+P+CARRYIN
      | B*(D-ACIN)+P+P
      | B*(D-ACIN)+P+P+CARRYIN
      | B*(D-ACIN)+P+P>>17
      | B*(D-ACIN)+P+P>>17+CARRYIN
      | B*(D-ACIN)+P+PCIN
      | B*(D-ACIN)+P+PCIN+CARRYIN
      | B*(D-ACIN)+P+PCIN>>17
      | B*(D-ACIN)+P+PCIN>>17+CARRYIN
      | B*(D-ACIN)+P-C
      | B*(D-ACIN)+P-P
      | B*(D-ACIN)+P-P>>17
      | B*(D-ACIN)+P-PCIN
      | B*(D-ACIN)+P-PCIN>>17
      | B*(D-ACIN)+P>>17
      | B*(D-ACIN)+P>>17+C
      | B*(D-ACIN)+P>>17+C+CARRYIN
      | B*(D-ACIN)+P>>17+CARRYIN
      | B*(D-ACIN)+P>>17+P
      | B*(D-ACIN)+P>>17+P+CARRYIN
      | B*(D-ACIN)+PCIN
      | B*(D-ACIN)+PCIN+C
      | B*(D-ACIN)+PCIN+C+CARRYIN
      | B*(D-ACIN)+PCIN+CARRYIN
      | B*(D-ACIN)+PCIN+P
      | B*(D-ACIN)+PCIN+P+CARRYIN
      | B*(D-ACIN)+PCIN>>17
      | B*(D-ACIN)+PCIN>>17+C
      | B*(D-ACIN)+PCIN>>17+C+CARRYIN
      | B*(D-ACIN)+PCIN>>17+CARRYIN
      | B*(D-ACIN)+PCIN>>17+P
      | B*(D-ACIN)+PCIN>>17+P+CARRYIN
      | B*(D-ACIN)-C
      | B*(D-ACIN)-P
      | B*(D-ACIN)-P>>17
      | B*(D-ACIN)-PCIN
      | B*(D-ACIN)-PCIN>>17
      | B*A
      | B*A+C
      | B*A+C+C
      | B*A+C+C+CARRYIN
      | B*A+C+CARRYIN
      | B*A+C+P
      | B*A+C+P+CARRYIN
      | B*A+C+P>>17
      | B*A+C+P>>17+CARRYIN
      | B*A+C+PCIN
      | B*A+C+PCIN+CARRYIN
      | B*A+C+PCIN>>17
      | B*A+C+PCIN>>17+CARRYIN
      | B*A+C-C
      | B*A+C-P
      | B*A+C-P>>17
      | B*A+C-PCIN
      | B*A+C-PCIN>>17
      | B*A+CARRYIN
      | B*A+P
      | B*A+P+C
      | B*A+P+C+CARRYIN
      | B*A+P+CARRYIN
      | B*A+P+P
      | B*A+P+P+CARRYIN
      | B*A+P+P>>17
      | B*A+P+P>>17+CARRYIN
      | B*A+P+PCIN
      | B*A+P+PCIN+CARRYIN
      | B*A+P+PCIN>>17
      | B*A+P+PCIN>>17+CARRYIN
      | B*A+P-C
      | B*A+P-P
      | B*A+P-P>>17
      | B*A+P-PCIN
      | B*A+P-PCIN>>17
      | B*A+P>>17
      | B*A+P>>17+C
      | B*A+P>>17+C+CARRYIN
      | B*A+P>>17+CARRYIN
      | B*A+P>>17+P
      | B*A+P>>17+P+CARRYIN
      | B*A+PCIN
      | B*A+PCIN+C
      | B*A+PCIN+C+CARRYIN
      | B*A+PCIN+CARRYIN
      | B*A+PCIN+P
      | B*A+PCIN+P+CARRYIN
      | B*A+PCIN>>17
      | B*A+PCIN>>17+C
      | B*A+PCIN>>17+C+CARRYIN
      | B*A+PCIN>>17+CARRYIN
      | B*A+PCIN>>17+P
      | B*A+PCIN>>17+P+CARRYIN
      | B*A-C
      | B*A-P
      | B*A-P>>17
      | B*A-PCIN
      | B*A-PCIN>>17
      | B*ACIN
      | B*ACIN+C
      | B*ACIN+C+C
      | B*ACIN+C+C+CARRYIN
      | B*ACIN+C+CARRYIN
      | B*ACIN+C+P
      | B*ACIN+C+P+CARRYIN
      | B*ACIN+C+P>>17
      | B*ACIN+C+P>>17+CARRYIN
      | B*ACIN+C+PCIN
      | B*ACIN+C+PCIN+CARRYIN
      | B*ACIN+C+PCIN>>17
      | B*ACIN+C+PCIN>>17+CARRYIN
      | B*ACIN+C-C
      | B*ACIN+C-P
      | B*ACIN+C-P>>17
      | B*ACIN+C-PCIN
      | B*ACIN+C-PCIN>>17
      | B*ACIN+CARRYIN
      | B*ACIN+P
      | B*ACIN+P+C
      | B*ACIN+P+C+CARRYIN
      | B*ACIN+P+CARRYIN
      | B*ACIN+P+P
      | B*ACIN+P+P+CARRYIN
      | B*ACIN+P+P>>17
      | B*ACIN+P+P>>17+CARRYIN
      | B*ACIN+P+PCIN
      | B*ACIN+P+PCIN+CARRYIN
      | B*ACIN+P+PCIN>>17
      | B*ACIN+P+PCIN>>17+CARRYIN
      | B*ACIN+P-C
      | B*ACIN+P-P
      | B*ACIN+P-P>>17
      | B*ACIN+P-PCIN
      | B*ACIN+P-PCIN>>17
      | B*ACIN+P>>17
      | B*ACIN+P>>17+C
      | B*ACIN+P>>17+C+CARRYIN
      | B*ACIN+P>>17+CARRYIN
      | B*ACIN+P>>17+P
      | B*ACIN+P>>17+P+CARRYIN
      | B*ACIN+PCIN
      | B*ACIN+PCIN+C
      | B*ACIN+PCIN+C+CARRYIN
      | B*ACIN+PCIN+CARRYIN
      | B*ACIN+PCIN+P
      | B*ACIN+PCIN+P+CARRYIN
      | B*ACIN+PCIN>>17
      | B*ACIN+PCIN>>17+C
      | B*ACIN+PCIN>>17+C+CARRYIN
      | B*ACIN+PCIN>>17+CARRYIN
      | B*ACIN+PCIN>>17+P
      | B*ACIN+PCIN>>17+P+CARRYIN
      | B*ACIN-C
      | B*ACIN-P
      | B*ACIN-P>>17
      | B*ACIN-PCIN
      | B*ACIN-PCIN>>17
      | B*D
      | B*D+C
      | B*D+C+C
      | B*D+C+C+CARRYIN
      | B*D+C+CARRYIN
      | B*D+C+P
      | B*D+C+P+CARRYIN
      | B*D+C+P>>17
      | B*D+C+P>>17+CARRYIN
      | B*D+C+PCIN
      | B*D+C+PCIN+CARRYIN
      | B*D+C+PCIN>>17
      | B*D+C+PCIN>>17+CARRYIN
      | B*D+C-C
      | B*D+C-P
      | B*D+C-P>>17
      | B*D+C-PCIN
      | B*D+C-PCIN>>17
      | B*D+CARRYIN
      | B*D+P
      | B*D+P+C
      | B*D+P+C+CARRYIN
      | B*D+P+CARRYIN
      | B*D+P+P
      | B*D+P+P+CARRYIN
      | B*D+P+P>>17
      | B*D+P+P>>17+CARRYIN
      | B*D+P+PCIN
      | B*D+P+PCIN+CARRYIN
      | B*D+P+PCIN>>17
      | B*D+P+PCIN>>17+CARRYIN
      | B*D+P-C
      | B*D+P-P
      | B*D+P-P>>17
      | B*D+P-PCIN
      | B*D+P-PCIN>>17
      | B*D+P>>17
      | B*D+P>>17+C
      | B*D+P>>17+C+CARRYIN
      | B*D+P>>17+CARRYIN
      | B*D+P>>17+P
      | B*D+P>>17+P+CARRYIN
      | B*D+PCIN
      | B*D+PCIN+C
      | B*D+PCIN+C+CARRYIN
      | B*D+PCIN+CARRYIN
      | B*D+PCIN+P
      | B*D+PCIN+P+CARRYIN
      | B*D+PCIN>>17
      | B*D+PCIN>>17+C
      | B*D+PCIN>>17+C+CARRYIN
      | B*D+PCIN>>17+CARRYIN
      | B*D+PCIN>>17+P
      | B*D+PCIN>>17+P+CARRYIN
      | B*D-C
      | B*D-P
      | B*D-P>>17
      | B*D-PCIN
      | B*D-PCIN>>17
      | BCIN*(A+D)
      | BCIN*(A+D)+C
      | BCIN*(A+D)+C+C
      | BCIN*(A+D)+C+C+CARRYIN
      | BCIN*(A+D)+C+CARRYIN
      | BCIN*(A+D)+C+P
      | BCIN*(A+D)+C+P+CARRYIN
      | BCIN*(A+D)+C+P>>17
      | BCIN*(A+D)+C+P>>17+CARRYIN
      | BCIN*(A+D)+C+PCIN
      | BCIN*(A+D)+C+PCIN+CARRYIN
      | BCIN*(A+D)+C+PCIN>>17
      | BCIN*(A+D)+C+PCIN>>17+CARRYIN
      | BCIN*(A+D)+C-C
      | BCIN*(A+D)+C-P
      | BCIN*(A+D)+C-P>>17
      | BCIN*(A+D)+C-PCIN
      | BCIN*(A+D)+C-PCIN>>17
      | BCIN*(A+D)+CARRYIN
      | BCIN*(A+D)+P
      | BCIN*(A+D)+P+C
      | BCIN*(A+D)+P+C+CARRYIN
      | BCIN*(A+D)+P+CARRYIN
      | BCIN*(A+D)+P+P
      | BCIN*(A+D)+P+P+CARRYIN
      | BCIN*(A+D)+P+P>>17
      | BCIN*(A+D)+P+P>>17+CARRYIN
      | BCIN*(A+D)+P+PCIN
      | BCIN*(A+D)+P+PCIN+CARRYIN
      | BCIN*(A+D)+P+PCIN>>17
      | BCIN*(A+D)+P+PCIN>>17+CARRYIN
      | BCIN*(A+D)+P-C
      | BCIN*(A+D)+P-P
      | BCIN*(A+D)+P-P>>17
      | BCIN*(A+D)+P-PCIN
      | BCIN*(A+D)+P-PCIN>>17
      | BCIN*(A+D)+P>>17
      | BCIN*(A+D)+P>>17+C
      | BCIN*(A+D)+P>>17+C+CARRYIN
      | BCIN*(A+D)+P>>17+CARRYIN
      | BCIN*(A+D)+P>>17+P
      | BCIN*(A+D)+P>>17+P+CARRYIN
      | BCIN*(A+D)+PCIN
      | BCIN*(A+D)+PCIN+C
      | BCIN*(A+D)+PCIN+C+CARRYIN
      | BCIN*(A+D)+PCIN+CARRYIN
      | BCIN*(A+D)+PCIN+P
      | BCIN*(A+D)+PCIN+P+CARRYIN
      | BCIN*(A+D)+PCIN>>17
      | BCIN*(A+D)+PCIN>>17+C
      | BCIN*(A+D)+PCIN>>17+C+CARRYIN
      | BCIN*(A+D)+PCIN>>17+CARRYIN
      | BCIN*(A+D)+PCIN>>17+P
      | BCIN*(A+D)+PCIN>>17+P+CARRYIN
      | BCIN*(A+D)-C
      | BCIN*(A+D)-P
      | BCIN*(A+D)-P>>17
      | BCIN*(A+D)-PCIN
      | BCIN*(A+D)-PCIN>>17
      | BCIN*(ACIN+D)
      | BCIN*(ACIN+D)+C
      | BCIN*(ACIN+D)+C+C
      | BCIN*(ACIN+D)+C+C+CARRYIN
      | BCIN*(ACIN+D)+C+CARRYIN
      | BCIN*(ACIN+D)+C+P
      | BCIN*(ACIN+D)+C+P+CARRYIN
      | BCIN*(ACIN+D)+C+P>>17
      | BCIN*(ACIN+D)+C+P>>17+CARRYIN
      | BCIN*(ACIN+D)+C+PCIN
      | BCIN*(ACIN+D)+C+PCIN+CARRYIN
      | BCIN*(ACIN+D)+C+PCIN>>17
      | BCIN*(ACIN+D)+C+PCIN>>17+CARRYIN
      | BCIN*(ACIN+D)+C-C
      | BCIN*(ACIN+D)+C-P
      | BCIN*(ACIN+D)+C-P>>17
      | BCIN*(ACIN+D)+C-PCIN
      | BCIN*(ACIN+D)+C-PCIN>>17
      | BCIN*(ACIN+D)+CARRYIN
      | BCIN*(ACIN+D)+P
      | BCIN*(ACIN+D)+P+C
      | BCIN*(ACIN+D)+P+C+CARRYIN
      | BCIN*(ACIN+D)+P+CARRYIN
      | BCIN*(ACIN+D)+P+P
      | BCIN*(ACIN+D)+P+P+CARRYIN
      | BCIN*(ACIN+D)+P+P>>17
      | BCIN*(ACIN+D)+P+P>>17+CARRYIN
      | BCIN*(ACIN+D)+P+PCIN
      | BCIN*(ACIN+D)+P+PCIN+CARRYIN
      | BCIN*(ACIN+D)+P+PCIN>>17
      | BCIN*(ACIN+D)+P+PCIN>>17+CARRYIN
      | BCIN*(ACIN+D)+P-C
      | BCIN*(ACIN+D)+P-P
      | BCIN*(ACIN+D)+P-P>>17
      | BCIN*(ACIN+D)+P-PCIN
      | BCIN*(ACIN+D)+P-PCIN>>17
      | BCIN*(ACIN+D)+P>>17
      | BCIN*(ACIN+D)+P>>17+C
      | BCIN*(ACIN+D)+P>>17+C+CARRYIN
      | BCIN*(ACIN+D)+P>>17+CARRYIN
      | BCIN*(ACIN+D)+P>>17+P
      | BCIN*(ACIN+D)+P>>17+P+CARRYIN
      | BCIN*(ACIN+D)+PCIN
      | BCIN*(ACIN+D)+PCIN+C
      | BCIN*(ACIN+D)+PCIN+C+CARRYIN
      | BCIN*(ACIN+D)+PCIN+CARRYIN
      | BCIN*(ACIN+D)+PCIN+P
      | BCIN*(ACIN+D)+PCIN+P+CARRYIN
      | BCIN*(ACIN+D)+PCIN>>17
      | BCIN*(ACIN+D)+PCIN>>17+C
      | BCIN*(ACIN+D)+PCIN>>17+C+CARRYIN
      | BCIN*(ACIN+D)+PCIN>>17+CARRYIN
      | BCIN*(ACIN+D)+PCIN>>17+P
      | BCIN*(ACIN+D)+PCIN>>17+P+CARRYIN
      | BCIN*(ACIN+D)-C
      | BCIN*(ACIN+D)-P
      | BCIN*(ACIN+D)-P>>17
      | BCIN*(ACIN+D)-PCIN
      | BCIN*(ACIN+D)-PCIN>>17
      | BCIN*(D+A)
      | BCIN*(D+A)+C
      | BCIN*(D+A)+C+C
      | BCIN*(D+A)+C+C+CARRYIN
      | BCIN*(D+A)+C+CARRYIN
      | BCIN*(D+A)+C+P
      | BCIN*(D+A)+C+P+CARRYIN
      | BCIN*(D+A)+C+P>>17
      | BCIN*(D+A)+C+P>>17+CARRYIN
      | BCIN*(D+A)+C+PCIN
      | BCIN*(D+A)+C+PCIN+CARRYIN
      | BCIN*(D+A)+C+PCIN>>17
      | BCIN*(D+A)+C+PCIN>>17+CARRYIN
      | BCIN*(D+A)+C-C
      | BCIN*(D+A)+C-P
      | BCIN*(D+A)+C-P>>17
      | BCIN*(D+A)+C-PCIN
      | BCIN*(D+A)+C-PCIN>>17
      | BCIN*(D+A)+CARRYIN
      | BCIN*(D+A)+P
      | BCIN*(D+A)+P+C
      | BCIN*(D+A)+P+C+CARRYIN
      | BCIN*(D+A)+P+CARRYIN
      | BCIN*(D+A)+P+P
      | BCIN*(D+A)+P+P+CARRYIN
      | BCIN*(D+A)+P+P>>17
      | BCIN*(D+A)+P+P>>17+CARRYIN
      | BCIN*(D+A)+P+PCIN
      | BCIN*(D+A)+P+PCIN+CARRYIN
      | BCIN*(D+A)+P+PCIN>>17
      | BCIN*(D+A)+P+PCIN>>17+CARRYIN
      | BCIN*(D+A)+P-C
      | BCIN*(D+A)+P-P
      | BCIN*(D+A)+P-P>>17
      | BCIN*(D+A)+P-PCIN
      | BCIN*(D+A)+P-PCIN>>17
      | BCIN*(D+A)+P>>17
      | BCIN*(D+A)+P>>17+C
      | BCIN*(D+A)+P>>17+C+CARRYIN
      | BCIN*(D+A)+P>>17+CARRYIN
      | BCIN*(D+A)+P>>17+P
      | BCIN*(D+A)+P>>17+P+CARRYIN
      | BCIN*(D+A)+PCIN
      | BCIN*(D+A)+PCIN+C
      | BCIN*(D+A)+PCIN+C+CARRYIN
      | BCIN*(D+A)+PCIN+CARRYIN
      | BCIN*(D+A)+PCIN+P
      | BCIN*(D+A)+PCIN+P+CARRYIN
      | BCIN*(D+A)+PCIN>>17
      | BCIN*(D+A)+PCIN>>17+C
      | BCIN*(D+A)+PCIN>>17+C+CARRYIN
      | BCIN*(D+A)+PCIN>>17+CARRYIN
      | BCIN*(D+A)+PCIN>>17+P
      | BCIN*(D+A)+PCIN>>17+P+CARRYIN
      | BCIN*(D+A)-C
      | BCIN*(D+A)-P
      | BCIN*(D+A)-P>>17
      | BCIN*(D+A)-PCIN
      | BCIN*(D+A)-PCIN>>17
      | BCIN*(D+ACIN)
      | BCIN*(D+ACIN)+C
      | BCIN*(D+ACIN)+C+C
      | BCIN*(D+ACIN)+C+C+CARRYIN
      | BCIN*(D+ACIN)+C+CARRYIN
      | BCIN*(D+ACIN)+C+P
      | BCIN*(D+ACIN)+C+P+CARRYIN
      | BCIN*(D+ACIN)+C+P>>17
      | BCIN*(D+ACIN)+C+P>>17+CARRYIN
      | BCIN*(D+ACIN)+C+PCIN
      | BCIN*(D+ACIN)+C+PCIN+CARRYIN
      | BCIN*(D+ACIN)+C+PCIN>>17
      | BCIN*(D+ACIN)+C+PCIN>>17+CARRYIN
      | BCIN*(D+ACIN)+C-C
      | BCIN*(D+ACIN)+C-P
      | BCIN*(D+ACIN)+C-P>>17
      | BCIN*(D+ACIN)+C-PCIN
      | BCIN*(D+ACIN)+C-PCIN>>17
      | BCIN*(D+ACIN)+CARRYIN
      | BCIN*(D+ACIN)+P
      | BCIN*(D+ACIN)+P+C
      | BCIN*(D+ACIN)+P+C+CARRYIN
      | BCIN*(D+ACIN)+P+CARRYIN
      | BCIN*(D+ACIN)+P+P
      | BCIN*(D+ACIN)+P+P+CARRYIN
      | BCIN*(D+ACIN)+P+P>>17
      | BCIN*(D+ACIN)+P+P>>17+CARRYIN
      | BCIN*(D+ACIN)+P+PCIN
      | BCIN*(D+ACIN)+P+PCIN+CARRYIN
      | BCIN*(D+ACIN)+P+PCIN>>17
      | BCIN*(D+ACIN)+P+PCIN>>17+CARRYIN
      | BCIN*(D+ACIN)+P-C
      | BCIN*(D+ACIN)+P-P
      | BCIN*(D+ACIN)+P-P>>17
      | BCIN*(D+ACIN)+P-PCIN
      | BCIN*(D+ACIN)+P-PCIN>>17
      | BCIN*(D+ACIN)+P>>17
      | BCIN*(D+ACIN)+P>>17+C
      | BCIN*(D+ACIN)+P>>17+C+CARRYIN
      | BCIN*(D+ACIN)+P>>17+CARRYIN
      | BCIN*(D+ACIN)+P>>17+P
      | BCIN*(D+ACIN)+P>>17+P+CARRYIN
      | BCIN*(D+ACIN)+PCIN
      | BCIN*(D+ACIN)+PCIN+C
      | BCIN*(D+ACIN)+PCIN+C+CARRYIN
      | BCIN*(D+ACIN)+PCIN+CARRYIN
      | BCIN*(D+ACIN)+PCIN+P
      | BCIN*(D+ACIN)+PCIN+P+CARRYIN
      | BCIN*(D+ACIN)+PCIN>>17
      | BCIN*(D+ACIN)+PCIN>>17+C
      | BCIN*(D+ACIN)+PCIN>>17+C+CARRYIN
      | BCIN*(D+ACIN)+PCIN>>17+CARRYIN
      | BCIN*(D+ACIN)+PCIN>>17+P
      | BCIN*(D+ACIN)+PCIN>>17+P+CARRYIN
      | BCIN*(D+ACIN)-C
      | BCIN*(D+ACIN)-P
      | BCIN*(D+ACIN)-P>>17
      | BCIN*(D+ACIN)-PCIN
      | BCIN*(D+ACIN)-PCIN>>17
      | BCIN*(D-A)
      | BCIN*(D-A)+C
      | BCIN*(D-A)+C+C
      | BCIN*(D-A)+C+C+CARRYIN
      | BCIN*(D-A)+C+CARRYIN
      | BCIN*(D-A)+C+P
      | BCIN*(D-A)+C+P+CARRYIN
      | BCIN*(D-A)+C+P>>17
      | BCIN*(D-A)+C+P>>17+CARRYIN
      | BCIN*(D-A)+C+PCIN
      | BCIN*(D-A)+C+PCIN+CARRYIN
      | BCIN*(D-A)+C+PCIN>>17
      | BCIN*(D-A)+C+PCIN>>17+CARRYIN
      | BCIN*(D-A)+C-C
      | BCIN*(D-A)+C-P
      | BCIN*(D-A)+C-P>>17
      | BCIN*(D-A)+C-PCIN
      | BCIN*(D-A)+C-PCIN>>17
      | BCIN*(D-A)+CARRYIN
      | BCIN*(D-A)+P
      | BCIN*(D-A)+P+C
      | BCIN*(D-A)+P+C+CARRYIN
      | BCIN*(D-A)+P+CARRYIN
      | BCIN*(D-A)+P+P
      | BCIN*(D-A)+P+P+CARRYIN
      | BCIN*(D-A)+P+P>>17
      | BCIN*(D-A)+P+P>>17+CARRYIN
      | BCIN*(D-A)+P+PCIN
      | BCIN*(D-A)+P+PCIN+CARRYIN
      | BCIN*(D-A)+P+PCIN>>17
      | BCIN*(D-A)+P+PCIN>>17+CARRYIN
      | BCIN*(D-A)+P-C
      | BCIN*(D-A)+P-P
      | BCIN*(D-A)+P-P>>17
      | BCIN*(D-A)+P-PCIN
      | BCIN*(D-A)+P-PCIN>>17
      | BCIN*(D-A)+P>>17
      | BCIN*(D-A)+P>>17+C
      | BCIN*(D-A)+P>>17+C+CARRYIN
      | BCIN*(D-A)+P>>17+CARRYIN
      | BCIN*(D-A)+P>>17+P
      | BCIN*(D-A)+P>>17+P+CARRYIN
      | BCIN*(D-A)+PCIN
      | BCIN*(D-A)+PCIN+C
      | BCIN*(D-A)+PCIN+C+CARRYIN
      | BCIN*(D-A)+PCIN+CARRYIN
      | BCIN*(D-A)+PCIN+P
      | BCIN*(D-A)+PCIN+P+CARRYIN
      | BCIN*(D-A)+PCIN>>17
      | BCIN*(D-A)+PCIN>>17+C
      | BCIN*(D-A)+PCIN>>17+C+CARRYIN
      | BCIN*(D-A)+PCIN>>17+CARRYIN
      | BCIN*(D-A)+PCIN>>17+P
      | BCIN*(D-A)+PCIN>>17+P+CARRYIN
      | BCIN*(D-A)-C
      | BCIN*(D-A)-P
      | BCIN*(D-A)-P>>17
      | BCIN*(D-A)-PCIN
      | BCIN*(D-A)-PCIN>>17
      | BCIN*(D-ACIN)
      | BCIN*(D-ACIN)+C
      | BCIN*(D-ACIN)+C+C
      | BCIN*(D-ACIN)+C+C+CARRYIN
      | BCIN*(D-ACIN)+C+CARRYIN
      | BCIN*(D-ACIN)+C+P
      | BCIN*(D-ACIN)+C+P+CARRYIN
      | BCIN*(D-ACIN)+C+P>>17
      | BCIN*(D-ACIN)+C+P>>17+CARRYIN
      | BCIN*(D-ACIN)+C+PCIN
      | BCIN*(D-ACIN)+C+PCIN+CARRYIN
      | BCIN*(D-ACIN)+C+PCIN>>17
      | BCIN*(D-ACIN)+C+PCIN>>17+CARRYIN
      | BCIN*(D-ACIN)+C-C
      | BCIN*(D-ACIN)+C-P
      | BCIN*(D-ACIN)+C-P>>17
      | BCIN*(D-ACIN)+C-PCIN
      | BCIN*(D-ACIN)+C-PCIN>>17
      | BCIN*(D-ACIN)+CARRYIN
      | BCIN*(D-ACIN)+P
      | BCIN*(D-ACIN)+P+C
      | BCIN*(D-ACIN)+P+C+CARRYIN
      | BCIN*(D-ACIN)+P+CARRYIN
      | BCIN*(D-ACIN)+P+P
      | BCIN*(D-ACIN)+P+P+CARRYIN
      | BCIN*(D-ACIN)+P+P>>17
      | BCIN*(D-ACIN)+P+P>>17+CARRYIN
      | BCIN*(D-ACIN)+P+PCIN
      | BCIN*(D-ACIN)+P+PCIN+CARRYIN
      | BCIN*(D-ACIN)+P+PCIN>>17
      | BCIN*(D-ACIN)+P+PCIN>>17+CARRYIN
      | BCIN*(D-ACIN)+P-C
      | BCIN*(D-ACIN)+P-P
      | BCIN*(D-ACIN)+P-P>>17
      | BCIN*(D-ACIN)+P-PCIN
      | BCIN*(D-ACIN)+P-PCIN>>17
      | BCIN*(D-ACIN)+P>>17
      | BCIN*(D-ACIN)+P>>17+C
      | BCIN*(D-ACIN)+P>>17+C+CARRYIN
      | BCIN*(D-ACIN)+P>>17+CARRYIN
      | BCIN*(D-ACIN)+P>>17+P
      | BCIN*(D-ACIN)+P>>17+P+CARRYIN
      | BCIN*(D-ACIN)+PCIN
      | BCIN*(D-ACIN)+PCIN+C
      | BCIN*(D-ACIN)+PCIN+C+CARRYIN
      | BCIN*(D-ACIN)+PCIN+CARRYIN
      | BCIN*(D-ACIN)+PCIN+P
      | BCIN*(D-ACIN)+PCIN+P+CARRYIN
      | BCIN*(D-ACIN)+PCIN>>17
      | BCIN*(D-ACIN)+PCIN>>17+C
      | BCIN*(D-ACIN)+PCIN>>17+C+CARRYIN
      | BCIN*(D-ACIN)+PCIN>>17+CARRYIN
      | BCIN*(D-ACIN)+PCIN>>17+P
      | BCIN*(D-ACIN)+PCIN>>17+P+CARRYIN
      | BCIN*(D-ACIN)-C
      | BCIN*(D-ACIN)-P
      | BCIN*(D-ACIN)-P>>17
      | BCIN*(D-ACIN)-PCIN